simulink model "hw3_control_DCM_constant_flux"  (R2022a)
settings: Solver=ode23t  StartTime=0.0  StopTime=12  FixedStep=0.00001  MaxStep=0.0001  RelTol=1e-3  EnableMultiTasking=on
values from neighbouring files (symbols this model uses):
  Fv = 0.01 + N/3000    (= 0.0236666666667)    [hw2.m]    (also in hw3.m)
  J = 0.46    [hw2.m]    (also in hw3.m)
  k1 = (kTI*J)/(2*TsumO*kfi*kTO)    (= 9.00740106793)    [hw3.m]
  k2 = (kTI*J)/(8*(TsumO^2)*kfi*kTO)    (= 1125.92513349)    [hw3.m]
  k3 = (Ta*Ra)/(2*TsumI*kD*kTI)    (= 0.356666666667)    [hw3.m]
  k4 = Ra/(2*TsumI*kD*kTI)    (= 294.504761905)    [hw3.m]
  kfi = M/In    (= 4.56074766355)    [hw2.m]    (also in hw3.m)
  kTI = 10/IAmax    (= 0.0467289719626)    [hw3.m]
  kTO = 10/On    (= 0.130812281993)    [hw3.m]
  La = 0.007    [hw2.m]    (also in hw3.m = 0.0007)
  Ra = 0.578    [hw2.m]    (also in hw3.m)
undefined symbols (not defined in the file or in its folder): sigbuilder_block
libraries: simulink x1
settings Model: Creator=user  LastModifiedBy=geote
settings SolverCC: AlgebraicLoopSolver=TrustRegion
settings DataIOCC: LimitDataPoints=on  SaveFormat=Array  SignalLoggingSaveFormat=ModelDataLogs  ReturnWorkspaceOutputs=off  SignalLoggingName=sigsOut
settings OptimizationCC: ExpressionDepthLimit=2147483647  InitFltsAndDblsToZero=on  LifeSpan=inf
settings DebuggingCC: SaveWithParameterizedLinksMsg=none  UnderspecifiedInitializationDetection=Classic  MergeDetectMultiDrivingBlocksExec=none  SignalResolutionControl=TryResolveAllWithWarning  MultiTaskDSMMsg=warning  MultiTaskCondExecSysMsg=none  FcnCallInpInsideContextMsg=warning  UnconnectedInputMsg=warning  UnconnectedOutputMsg=warning  UnconnectedLineMsg=warning  StateNameClashWarn=warning  SFUnexpectedBacktrackingDiag=warning  SFNoUnconditionalDefaultTransitionDiag=warning  SFMachineParentedDataDiag=warning  ArithmeticOperatorsInVariantConditions=warning
settings HardwareCC: ProdBitPerPointer=32  ProdBitPerSizeT=32  ProdBitPerPtrDiffT=32  ProdLargestAtomicFloat=None  ProdIntDivRoundTo=Undefined  ProdEndianess=Unspecified  ProdWordSize=32  ProdHWDeviceType=32-bit Generic  TargetUnknown=on
settings ModelReferenceCC: PropagateSignalLabelsOutOfModel=off
settings SFSimCC: SimUseLocalCustomCode=off  CustomCodeUndefinedFunction=UseInterfaceOnly
settings RTWCC: RTWUseLocalCustomCode=off
settings CodeAppCC: StateflowObjectComments=on
settings GRTTargetCC: TargetFcnLib=ansi_tfl_tmw.mat  TargetLangStandard=C89/C90 (ANSI)  CombineOutputUpdateFcns=off  GRTInterface=on
settings ConfigComp: CovMetricSettings=dw  CovSaveCumulativeToWorkspaceVar=on  CovSaveSingleToWorkspaceVar=on  CovExternalEMLEnable=off

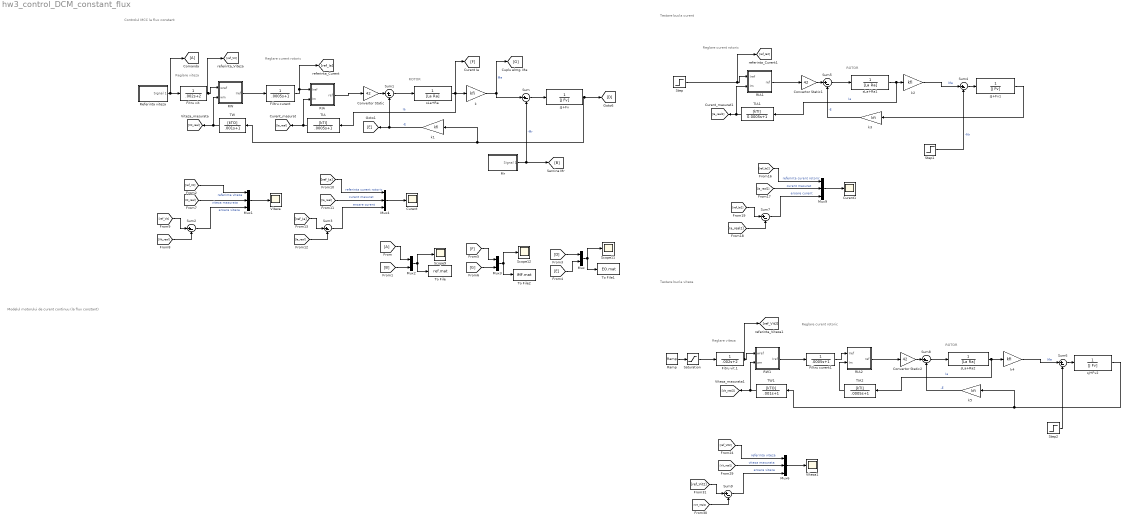
== system hw3_control_DCM_constant_flux ==
  note: Reglare viteza
  note: Reglare curent rotoric
  note: ROTOR
  note: Controlul MCC la flux constant
  note: Reglare curent rotoric
  note: ROTOR
  note: Testare bucla curent
  note: Reglare viteza
  note: Reglare curent rotoric
  note: ROTOR
  note: Testare bucla viteza
  note: Modelul motorului de curent continuu (la flux constant)
  Comanda : Goto
  Convertor Static : Gain  Gain=42  SaturateOnIntegerOverflow=on  OutDataTypeStr=Inherit: Same as input  ParamDataTypeStr=Inherit: Same as input
  Convertor Static1 : Gain  Gain=42  SaturateOnIntegerOverflow=on  OutDataTypeStr=Inherit: Same as input  ParamDataTypeStr=Inherit: Same as input
  Convertor Static2 : Gain  Gain=42  SaturateOnIntegerOverflow=on  OutDataTypeStr=Inherit: Same as input  ParamDataTypeStr=Inherit: Same as input
  Curent : Scope
  Curent1 : Scope
  Filtru curent : TransferFcn  Denominator=[.0005 1]
  Filtru curent1 : TransferFcn  Denominator=[.0005 1]
  Fitru vit. : TransferFcn  Denominator=[.002 2]
  Fitru vit.1 : TransferFcn  Denominator=[.002 2]
  From : From
      settings: CloseFcn=tagdialog Close
  Mr : Subsystem  Tag=STV Subsys  LoadFcn=sigbuilder_block('load');  StartFcn=sigbuilder_block('start');  StopFcn=sigbuilder_block('stop');  ClipboardFcn=sigbuilder_block('clipboard');  mask(Sigbuilder block){tuvar=[0:10]}
      mask help: Sigbuilder GUI
      mask init:
      | if ~strcmp(get_param(bdroot(gcbh),'SimulationStatus'),'stopped') tuvar = sigbuilder_block('maskInit'); end
      settings: CopyFcn=sigbuilder_block('copy');  DeleteFcn=sigbuilder_block('delete');  ModelCloseFcn=sigbuilder_block('modelClose');  PreSaveFcn=sigbuilder_block('preSave');  PauseFcn=sigbuilder_block('pause')  ContinueFcn=sigbuilder_block('continue')  NameChangeFcn=sigbuilder_block('namechange');  OpenFcn=sigbuilder_block('open',[279.75 230.25 550.5 362.25 ]);  CloseFcn=sigbuilder_block('close');
      mask Display: plot(0, 0, 100, 100,[2, 2, 32, 32, 2], [68, 8, 8, 68, 68],[32, 2], [38, 38], [32, 19, 2],[53, 60, 44], [32, 17, 17, 2],[16, 16, 31, 31]);txt = getActiveGroup(gcbh);text(2, 100, txt,'verticalAlignment', 'top');
  Mux : Mux  Inputs=2
  Mux{1,4,6,9} (4 alike) : Mux  Inputs=3
  Mux2 : Mux  Inputs=2
  Mux3 : Mux  Inputs=2
  RIA : Subsystem  mask(PI controller){Kp=k3, Ki=k4, Ilim=400}
      mask prompts: Kp "Proportional gain (Kp)"; Ki "Integral gain (Ki)"; Ilim "Current limit (A)"
      mask help: Proportional-Integral Speed Controller
      mask Display: plot(0,0,100,100,[35,35,65],[70,30,30],[35,65],[45,60])
  RIA1 : Subsystem  mask(PI controller){Kp=k3, Ki=k4, Ilim=214}
      mask prompts: Kp "Proportional gain (Kp)"; Ki "Integral gain (Ki)"; Ilim "Current limit (A)"
      mask help: Proportional-Integral Speed Controller
      mask Display: plot(0,0,100,100,[35,35,65],[70,30,30],[35,65],[45,60])
  RIA2 : Subsystem  mask(PI controller){Kp=3.5667, Ki=294.5048, Ilim=214}
      mask prompts: Kp "Proportional gain (Kp)"; Ki "Integral gain (Ki)"; Ilim "Current limit (A)"
      mask help: Proportional-Integral Speed Controller
      mask Display: plot(0,0,100,100,[35,35,65],[70,30,30],[35,65],[45,60])
  RW : Subsystem  mask(PI controller){Kp=k1, Ki=k2, Ilim=214}
      mask prompts: Kp "Proportional gain (Kp)"; Ki "Integral gain (Ki)"; Ilim "Current limit (A)"
      mask help: Proportional-Integral Speed Controller
      mask Display: plot(0,0,100,100,[35,35,65],[70,30,30],[35,65],[45,60])
  RW1 : Subsystem  mask(PI controller){Kp=k1, Ki=k2, Ilim=214}
      mask prompts: Kp "Proportional gain (Kp)"; Ki "Integral gain (Ki)"; Ilim "Current limit (A)"
      mask help: Proportional-Integral Speed Controller
      mask Display: plot(0,0,100,100,[35,35,65],[70,30,30],[35,65],[45,60])
  Ramp : simulink/Sources/Ramp  slope=50  start=1  InitialOutput=0
  Referinta viteza : Subsystem  Tag=STV Subsys  LoadFcn=sigbuilder_block('load');  StartFcn=sigbuilder_block('start');  StopFcn=sigbuilder_block('stop');  ClipboardFcn=sigbuilder_block('clipboard');  mask(Sigbuilder block){tuvar=[0:10]}
      mask help: Sigbuilder GUI
      mask init:
      | if ~strcmp(get_param(bdroot(gcbh),'SimulationStatus'),'stopped') tuvar = sigbuilder_block('maskInit'); end
      settings: CopyFcn=sigbuilder_block('copy');  DeleteFcn=sigbuilder_block('delete');  ModelCloseFcn=sigbuilder_block('modelClose');  PreSaveFcn=sigbuilder_block('preSave');  PauseFcn=sigbuilder_block('pause')  ContinueFcn=sigbuilder_block('continue')  NameChangeFcn=sigbuilder_block('namechange');  OpenFcn=sigbuilder_block('open',[300 102.6 550.8 362.4 ]);  CloseFcn=sigbuilder_block('close');
      mask Display: plot(0, 0, 100, 100,[2, 2, 32, 32, 2], [68, 8, 8, 68, 68],[32, 2], [38, 38], [32, 19, 2],[53, 60, 44], [32, 17, 17, 2],[16, 16, 31, 31]);txt = getActiveGroup(gcbh);text(2, 100, txt,'verticalAlignment', 'top');
  Saturation : Saturate  UpperLimit=10  LowerLimit=-10
  Scope11 : Scope
  Scope12 : Scope
  Scope9 : Scope
  Step : Step  After=10  SampleTime=0
  Step1 : Step  After=10  SampleTime=0
  Step2 : Step  Time=1.5  After=10  SampleTime=0
  Sum{,1..9} (10 alike) : Sum  Inputs=|+-  SaturateOnIntegerOverflow=on
  TIA : TransferFcn  Numerator=[kTI]  Denominator=[.0005 1]
  TIA1 : TransferFcn  Numerator=[kTI]  Denominator=[0.0005 1]
  TIA2 : TransferFcn  Numerator=[kTI]  Denominator=[.0005 1]
  TW : TransferFcn  Numerator=[kTO]  Denominator=[.001 1]
  TW1 : TransferFcn  Numerator=[kTO]  Denominator=[.001 1]
  To File : ToFile  Filename=ref.mat  MatrixName=ref
  To File1 : ToFile  Filename=EO.mat  MatrixName=EO
  To File2 : ToFile  Filename=IMF.mat  MatrixName=IMF
  Viteza : Scope
  Viteza1 : Scope
  k{,1..5} (6 alike) : Gain  Gain=kfi  SaturateOnIntegerOverflow=on  OutDataTypeStr=Inherit: Same as input  ParamDataTypeStr=Inherit: Same as input
  sJ+Fv : TransferFcn  Denominator=[J Fv]
  sJ+Fv1 : TransferFcn  Denominator=[J Fv]
  sJ+Fv2 : TransferFcn  Denominator=[J Fv]
  sLa+Ra : TransferFcn  Denominator=[La Ra]
  sLa+Ra1 : TransferFcn  Denominator=[La Ra]
  sLa+Ra2 : TransferFcn  Denominator=[La Ra]
  wires:
  Sum1/1 -> sLa+Ra/1
  sLa+Ra/1 -> TIA/1, k/1, [F]  "Ia"
  sJ+Fv/1 -> k1/1, TW/1, [D]
  k1/1 -> [E], Sum1/2  "-E"
  k/1 -> Sum/1, [G]  "Me"
  Sum/1 -> sJ+Fv/1
  Convertor Static/1 -> Sum1/1
  RIA/1(ref) -> Convertor Static/1
  TIA/1 -> [Ia_real], RIA/2(Im)
  TW/1 -> [Vit_real], RW/2(wm)
  Filtru curent/1 -> [ref_Ia], RIA/1(Iref)
  Fitru vit./1 -> [ref_Vit], RW/1(wref)
  RW/1(Iref) -> Filtru curent/1
  Mux/1 -> Scope11/1, To File1/1
  Mux3/1 -> Scope12/1, To File2/1
  Mux2/1 -> Scope9/1, To File/1
  From/1 -> Mux2/1
  [B] -> Mux2/2
  [D] -> Mux/1
  [E] -> Mux/2
  [F] -> Mux3/1
  [G] -> Mux3/2
  Referinta viteza/1(Signal 1) -> Fitru vit./1, Comanda/1
  Mr/1(Signal 1) -> [B], Sum/2  "-Mr"
  Mux1/1 -> Viteza/1
  [ref_Vit] -> Mux1/1  "referinta viteza"
  [Vit_real] -> Mux1/2  "viteza masurata"
  [ref_Vit] -> Sum2/1
  [Vit_real] -> Sum2/2
  Sum2/1 -> Mux1/3  "eroare viteza"
  Mux4/1 -> Curent/1
  [ref_Ia] -> Mux4/1  "referinta curent rotoric"
  [Ia_real] -> Mux4/2  "curent masurat"
  [ref_Ia] -> Sum3/1
  [Ia_real] -> Sum3/2
  Sum3/1 -> Mux4/3  "eroare curent"
  Sum5/1 -> sLa+Ra1/1
  sLa+Ra1/1 -> TIA1/1, k2/1  "Ia"
  sJ+Fv1/1 -> k3/1
  k3/1 -> Sum5/2  "-E"
  k2/1 -> Sum4/1  "Me"
  Sum4/1 -> sJ+Fv1/1
  Convertor Static1/1 -> Sum5/1
  Step/1 -> RIA1/1(Iref), [ref_Ia2]
  Step1/1 -> Sum4/2  "-Mr"
  Mux9/1 -> Curent1/1
  [ref_Ia2] -> Mux9/1  "referinta curent rotoric"
  [Ia_real2] -> Mux9/2  "curent masurat"
  [ref_Ia2] -> Sum7/1
  [Ia_real2] -> Sum7/2
  Sum7/1 -> Mux9/3  "eroare curent"
  Sum8/1 -> sLa+Ra2/1
  sLa+Ra2/1 -> k4/1, TIA2/1  "Ia"
  sJ+Fv2/1 -> TW1/1, k5/1
  k5/1 -> Sum8/2  "-E"
  k4/1 -> Sum6/1  "Me"
  Sum6/1 -> sJ+Fv2/1
  Convertor Static2/1 -> Sum8/1
  RIA2/1(ref) -> Convertor Static2/1
  TIA2/1 -> RIA2/2(Im)
  TW1/1 -> RW1/2(wm), [Vit_real2]
  Filtru curent1/1 -> RIA2/1(Iref)
  Fitru vit.1/1 -> [ref_Vit2], RW1/1(wref)
  RW1/1(Iref) -> Filtru curent1/1
  Saturation/1 -> Fitru vit.1/1
  Mux6/1 -> Viteza1/1
  [ref_Vit2] -> Mux6/1  "referinta viteza"
  [Vit_real2] -> Mux6/2  "viteza masurata"
  [ref_Vit2] -> Sum9/1
  [Vit_real2] -> Sum9/2
  Sum9/1 -> Mux6/3  "eroare viteza"
  Ramp/1 -> Saturation/1
  Step2/1 -> Sum6/2
  RIA1/1(ref) -> Convertor Static1/1
  TIA1/1 -> RIA1/2(Im), [Ia_real2]

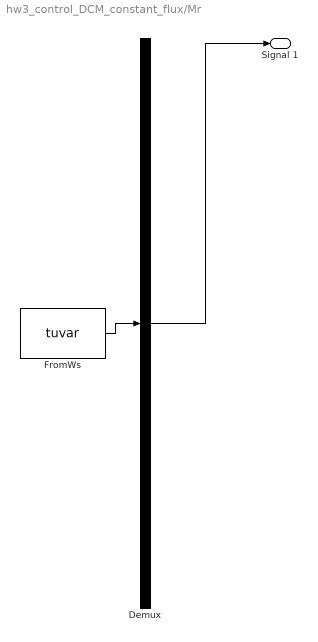
== system hw3_control_DCM_constant_flux/Mr ==
  Demux : Demux  Tag=STV Demux  Outputs=1
      settings: DisplayOption=none
  FromWs : FromWorkspace  Tag=STV FromWs  VariableName=tuvar
      SigBuilderData=|
      | common.afterFinalStr = 'Extrapolate'
      | common.sampleTime = 0
      | dataSet.timeRange = [0 10]
      | dataSet.name = 'Group 1'
      | sbobj.Groups.Name = 'Group 1'
      | sbobj.Groups.Signals.Name = 'Signal 1'
      | sbobj.Groups.Signals.XData = [0 1.5 1.9 5.5 7 10]
      | sbobj.Groups.Signals.YData = [0 0 12.47 12.47 -12.47 -12.47]
  Signal 1 : Outport  Tag=STV Outport
  wires:
  FromWs/1 -> Demux/1
  Demux/1 -> Signal 1/1

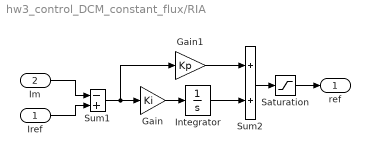
== system hw3_control_DCM_constant_flux/RIA ==
  Iref : Inport
  Im : Inport  Port=2
  Gain : Gain  Gain=Ki  SaturateOnIntegerOverflow=on  OutDataTypeStr=Inherit: Same as input  ParamDataTypeStr=Inherit: Same as input
  Gain1 : Gain  Gain=Kp  SaturateOnIntegerOverflow=on  OutDataTypeStr=Inherit: Same as input  ParamDataTypeStr=Inherit: Same as input
  Integrator : Integrator  LimitOutput=on  UpperSaturationLimit=Ilim  LowerSaturationLimit=-Ilim
  Saturation : Saturate  UpperLimit=Ilim  LowerLimit=-Ilim
  Sum1 : Sum  Inputs=-+  InputSameDT=on  SaturateOnIntegerOverflow=on  OutDataTypeStr=Inherit: Same as first input
  Sum2 : Sum  InputSameDT=on  SaturateOnIntegerOverflow=on  OutDataTypeStr=Inherit: Same as first input
  ref : Outport  InitialOutput=0
  wires:
  Saturation/1 -> ref/1
  Sum2/1 -> Saturation/1
  Integrator/1 -> Sum2/2
  Iref/1 -> Sum1/2
  Im/1 -> Sum1/1
  Sum1/1 -> Gain1/1, Gain/1
  Gain/1 -> Integrator/1
  Gain1/1 -> Sum2/1

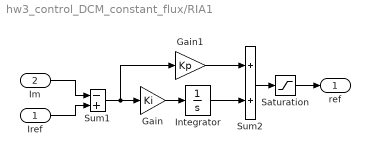
== system hw3_control_DCM_constant_flux/RIA1 ==
  Iref : Inport
  Im : Inport  Port=2
  Gain : Gain  Gain=Ki  SaturateOnIntegerOverflow=on  OutDataTypeStr=Inherit: Same as input  ParamDataTypeStr=Inherit: Same as input
  Gain1 : Gain  Gain=Kp  SaturateOnIntegerOverflow=on  OutDataTypeStr=Inherit: Same as input  ParamDataTypeStr=Inherit: Same as input
  Integrator : Integrator  LimitOutput=on  UpperSaturationLimit=Ilim  LowerSaturationLimit=-Ilim
  Saturation : Saturate  UpperLimit=Ilim  LowerLimit=-Ilim
  Sum1 : Sum  Inputs=-+  InputSameDT=on  SaturateOnIntegerOverflow=on  OutDataTypeStr=Inherit: Same as first input
  Sum2 : Sum  InputSameDT=on  SaturateOnIntegerOverflow=on  OutDataTypeStr=Inherit: Same as first input
  ref : Outport  InitialOutput=0
  wires:
  Gain1/1 -> Sum2/1
  Gain/1 -> Integrator/1
  Sum1/1 -> Gain/1, Gain1/1
  Im/1 -> Sum1/1
  Iref/1 -> Sum1/2
  Integrator/1 -> Sum2/2
  Sum2/1 -> Saturation/1
  Saturation/1 -> ref/1

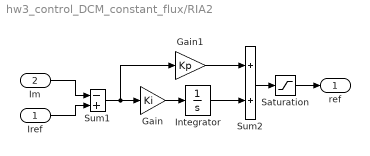
== system hw3_control_DCM_constant_flux/RIA2 ==
  Iref : Inport
  Im : Inport  Port=2
  Gain : Gain  Gain=Ki  SaturateOnIntegerOverflow=on  OutDataTypeStr=Inherit: Same as input  ParamDataTypeStr=Inherit: Same as input
  Gain1 : Gain  Gain=Kp  SaturateOnIntegerOverflow=on  OutDataTypeStr=Inherit: Same as input  ParamDataTypeStr=Inherit: Same as input
  Integrator : Integrator  LimitOutput=on  UpperSaturationLimit=Ilim  LowerSaturationLimit=-Ilim
  Saturation : Saturate  UpperLimit=Ilim  LowerLimit=-Ilim
  Sum1 : Sum  Inputs=-+  InputSameDT=on  SaturateOnIntegerOverflow=on  OutDataTypeStr=Inherit: Same as first input
  Sum2 : Sum  InputSameDT=on  SaturateOnIntegerOverflow=on  OutDataTypeStr=Inherit: Same as first input
  ref : Outport  InitialOutput=0
  wires:
  Gain1/1 -> Sum2/1
  Gain/1 -> Integrator/1
  Sum1/1 -> Gain/1, Gain1/1
  Im/1 -> Sum1/1
  Iref/1 -> Sum1/2
  Integrator/1 -> Sum2/2
  Sum2/1 -> Saturation/1
  Saturation/1 -> ref/1

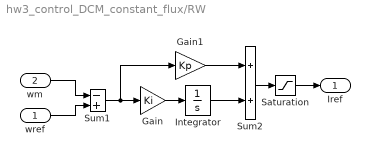
== system hw3_control_DCM_constant_flux/RW ==
  wref : Inport
  wm : Inport  Port=2
  Gain : Gain  Gain=Ki  SaturateOnIntegerOverflow=on  OutDataTypeStr=Inherit: Same as input  ParamDataTypeStr=Inherit: Same as input
  Gain1 : Gain  Gain=Kp  SaturateOnIntegerOverflow=on  OutDataTypeStr=Inherit: Same as input  ParamDataTypeStr=Inherit: Same as input
  Integrator : Integrator  LimitOutput=on  UpperSaturationLimit=Ilim  LowerSaturationLimit=-Ilim
  Saturation : Saturate  UpperLimit=Ilim  LowerLimit=-Ilim
  Sum1 : Sum  Inputs=-+  InputSameDT=on  SaturateOnIntegerOverflow=on  OutDataTypeStr=Inherit: Same as first input
  Sum2 : Sum  InputSameDT=on  SaturateOnIntegerOverflow=on  OutDataTypeStr=Inherit: Same as first input
  Iref : Outport  InitialOutput=0
  wires:
  Saturation/1 -> Iref/1
  Sum2/1 -> Saturation/1
  Integrator/1 -> Sum2/2
  wref/1 -> Sum1/2
  wm/1 -> Sum1/1
  Sum1/1 -> Gain1/1, Gain/1
  Gain/1 -> Integrator/1
  Gain1/1 -> Sum2/1

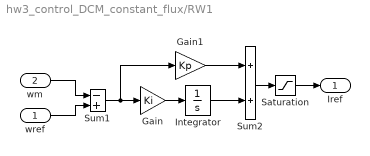
== system hw3_control_DCM_constant_flux/RW1 ==
  wref : Inport
  wm : Inport  Port=2
  Gain : Gain  Gain=Ki  SaturateOnIntegerOverflow=on  OutDataTypeStr=Inherit: Same as input  ParamDataTypeStr=Inherit: Same as input
  Gain1 : Gain  Gain=Kp  SaturateOnIntegerOverflow=on  OutDataTypeStr=Inherit: Same as input  ParamDataTypeStr=Inherit: Same as input
  Integrator : Integrator  LimitOutput=on  UpperSaturationLimit=Ilim  LowerSaturationLimit=-Ilim
  Saturation : Saturate  UpperLimit=Ilim  LowerLimit=-Ilim
  Sum1 : Sum  Inputs=-+  InputSameDT=on  SaturateOnIntegerOverflow=on  OutDataTypeStr=Inherit: Same as first input
  Sum2 : Sum  InputSameDT=on  SaturateOnIntegerOverflow=on  OutDataTypeStr=Inherit: Same as first input
  Iref : Outport  InitialOutput=0
  wires:
  Gain1/1 -> Sum2/1
  Gain/1 -> Integrator/1
  Sum1/1 -> Gain/1, Gain1/1
  wm/1 -> Sum1/1
  wref/1 -> Sum1/2
  Integrator/1 -> Sum2/2
  Sum2/1 -> Saturation/1
  Saturation/1 -> Iref/1

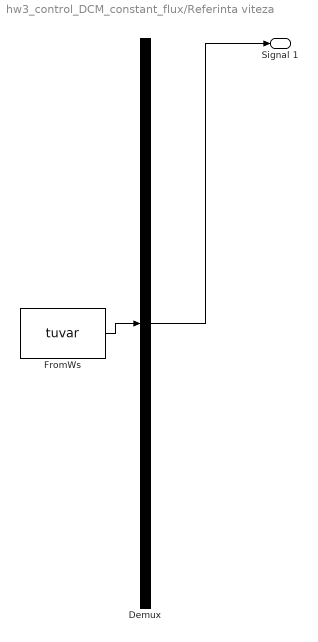
== system hw3_control_DCM_constant_flux/Referinta viteza ==
  Demux : Demux  Tag=STV Demux  Outputs=1
      settings: DisplayOption=none
  FromWs : FromWorkspace  Tag=STV FromWs  VariableName=tuvar
      SigBuilderData=|
      | common.afterFinalStr = 'Extrapolate'
      | common.sampleTime = 0
      | dataSet.timeRange = [0 10]
      | dataSet.name = 'Group 1'
      | sbobj.Groups.Name = 'Group 1'
      | sbobj.Groups.Signals.Name = 'Signal 1'
      | sbobj.Groups.Signals.XData = [0 1 2 5 7 10]
      | sbobj.Groups.Signals.YData = [0 0 10 10 -10 -10]
  Signal 1 : Outport  Tag=STV Outport
  wires:
  Demux/1 -> Signal 1/1
  FromWs/1 -> Demux/1
